simulink library "Garbage"  (R2023a)
settings: Solver=ode45  StartTime=0.0  StopTime=10.0  RelTol=1e-3  EnableMultiTasking=on
undefined symbols (not defined in the file or in its folder): c, ending_column_index, ending_row_index, framebuffer_16x1, framebuffer_2x2, framebuffer_4x4, framebuffer_8x8, HEIGHT, hot, POS_X, POS_Y, starting_column_index, starting_row_index, WIDTH
libraries: Memory_Blocks_Lib x459, simulink x235, simulink_extras x132, dspmtrx3 x116, Bit_Multiplexers_Lib x67, Bit_Demultiplexers_Lib x49, Byte_Multiplexers_Lib x40, Miscellaneous x38, Computer_Library x17, Decoders_Lib x14, commutil2 x12, Arithmetic_Operations_Lib x8
(part 3 of 26)

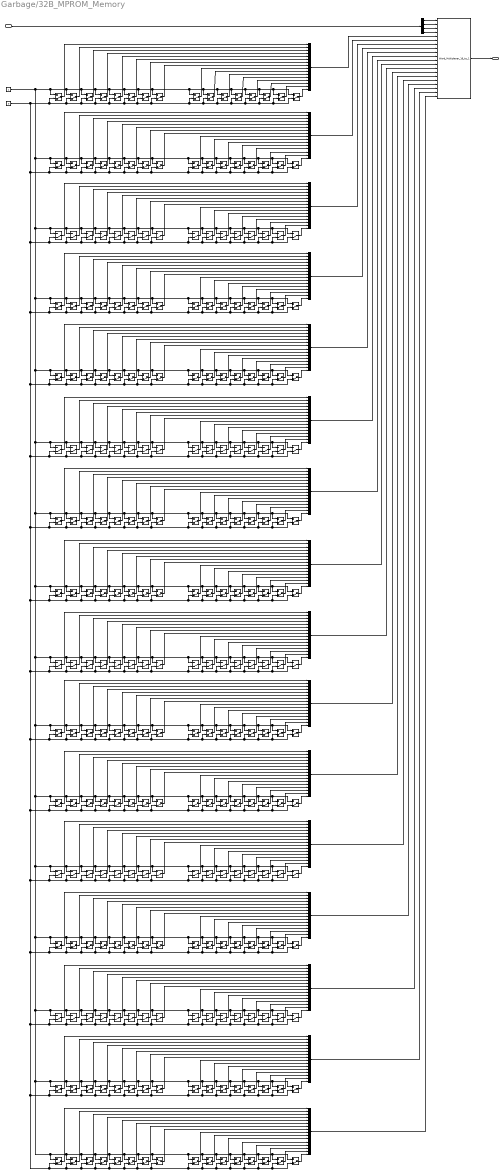
== system Garbage/32B_MPROM_Memory ==
  note: Manually Programmable Read Only Memory (MPROM)
  Addr(2B) : Inport
  Bus Creator{,1..15} (16 alike) : BusCreator  Inputs=16
  Bus Selector : BusSelector  OutputSignals=signal13,signal14,signal15,signal16
  Constant : Constant
  Constant1 : Constant  Value=0
  Manual Switch{,1..156,158..255} (255 alike) : ManualSwitch  CurrentSetting=0
  Manual Switch157 : ManualSwitch
  Word_Multiplexer_16_to_1 : Word_Multiplexers_Lib/Word_Multiplexers_Lib/Word_Multiplexer_16_to_1
  Data(2B) : Outport
  wires:
  Addr(2B)/1 -> Bus Selector/1
  Manual Switch54/1 -> Bus Creator3/16
  Manual Switch59/1 -> Bus Creator3/7
  Manual Switch50/1 -> Bus Creator3/12
  Manual Switch57/1 -> Bus Creator3/5
  Manual Switch53/1 -> Bus Creator3/15
  Manual Switch48/1 -> Bus Creator3/1
  Manual Switch61/1 -> Bus Creator3/9
  Manual Switch52/1 -> Bus Creator3/14
  Manual Switch51/1 -> Bus Creator3/13
  Manual Switch56/1 -> Bus Creator3/4
  Manual Switch49/1 -> Bus Creator3/3
  Manual Switch58/1 -> Bus Creator3/6
  Manual Switch63/1 -> Bus Creator3/11
  Manual Switch62/1 -> Bus Creator3/10
  Manual Switch60/1 -> Bus Creator3/8
  Manual Switch55/1 -> Bus Creator3/2
  Manual Switch14/1 -> Bus Creator/16
  Manual Switch4/1 -> Bus Creator/6
  Manual Switch5/1 -> Bus Creator/7
  Manual Switch3/1 -> Bus Creator/5
  Manual Switch/1 -> Bus Creator/1
  Manual Switch99/1 -> Bus Creator6/13
  Manual Switch98/1 -> Bus Creator6/12
  Manual Switch111/1 -> Bus Creator6/11
  Manual Switch100/1 -> Bus Creator6/14
  Manual Switch110/1 -> Bus Creator6/10
  Manual Switch108/1 -> Bus Creator6/8
  Manual Switch109/1 -> Bus Creator6/9
  Manual Switch102/1 -> Bus Creator6/16
  Manual Switch101/1 -> Bus Creator6/15
  Manual Switch107/1 -> Bus Creator6/7
  Manual Switch104/1 -> Bus Creator6/4
  Manual Switch97/1 -> Bus Creator6/3
  Manual Switch103/1 -> Bus Creator6/2
  Manual Switch96/1 -> Bus Creator6/1
  Manual Switch105/1 -> Bus Creator6/5
  Manual Switch106/1 -> Bus Creator6/6
  Manual Switch85/1 -> Bus Creator5/15
  Manual Switch83/1 -> Bus Creator5/13
  Manual Switch82/1 -> Bus Creator5/12
  Manual Switch86/1 -> Bus Creator5/16
  Manual Switch84/1 -> Bus Creator5/14
  Manual Switch93/1 -> Bus Creator5/9
  Manual Switch91/1 -> Bus Creator5/7
  Manual Switch95/1 -> Bus Creator5/11
  Manual Switch94/1 -> Bus Creator5/10
  Manual Switch92/1 -> Bus Creator5/8
  Manual Switch90/1 -> Bus Creator5/6
  Manual Switch89/1 -> Bus Creator5/5
  Manual Switch88/1 -> Bus Creator5/4
  Manual Switch81/1 -> Bus Creator5/3
  Manual Switch87/1 -> Bus Creator5/2
  Manual Switch80/1 -> Bus Creator5/1
  Manual Switch130/1 -> Bus Creator8/12
  Manual Switch245/1 -> Bus Creator8/11
  Manual Switch234/1 -> Bus Creator8/10
  Manual Switch168/1 -> Bus Creator8/4
  Manual Switch141/1 -> Bus Creator8/13
  Manual Switch128/1 -> Bus Creator8/1
  Manual Switch169/1 -> Bus Creator9/14
  Manual Switch167/1 -> Bus Creator9/13
  Manual Switch166/1 -> Bus Creator9/12
  Manual Switch129/1 -> Bus Creator8/3
  Manual Switch170/1 -> Bus Creator9/15
  Manual Switch162/1 -> Bus Creator8/16
  Manual Switch161/1 -> Bus Creator8/15
  Manual Switch163/1 -> Bus Creator8/2
  Manual Switch171/1 -> Bus Creator9/16
  Manual Switch179/1 -> Bus Creator8/5
  Manual Switch223/1 -> Bus Creator8/9
  Manual Switch212/1 -> Bus Creator8/8
  Manual Switch201/1 -> Bus Creator8/7
  Manual Switch152/1 -> Bus Creator8/14
  Manual Switch190/1 -> Bus Creator8/6
  Manual Switch186/1 -> Bus Creator10/14
  Manual Switch184/1 -> Bus Creator10/12
  Manual Switch197/1 -> Bus Creator10/10
  Manual Switch192/1 -> Bus Creator10/5
  Manual Switch211/1 -> Bus Creator11/7
  Manual Switch209/1 -> Bus Creator11/5
  Manual Switch187/1 -> Bus Creator10/15
  Manual Switch173/1 -> Bus Creator9/4
  Manual Switch195/1 -> Bus Creator10/8
  Manual Switch200/1 -> Bus Creator11/3
  Manual Switch216/1 -> Bus Creator11/11
  Manual Switch199/1 -> Bus Creator11/1
  Manual Switch178/1 -> Bus Creator9/9
  Manual Switch191/1 -> Bus Creator10/4
  Manual Switch214/1 -> Bus Creator11/9
  Manual Switch221/1 -> Bus Creator12/14
  Manual Switch220/1 -> Bus Creator12/13
  Manual Switch232/1 -> Bus Creator12/10
  Manual Switch213/1 -> Bus Creator11/8
  Manual Switch233/1 -> Bus Creator12/11
  Manual Switch231/1 -> Bus Creator12/9
  Manual Switch174/1 -> Bus Creator9/5
  Manual Switch177/1 -> Bus Creator9/8
  Manual Switch189/1 -> Bus Creator10/2
  Manual Switch180/1 -> Bus Creator9/10
  Manual Switch182/1 -> Bus Creator10/1
  Manual Switch203/1 -> Bus Creator11/13
  Manual Switch204/1 -> Bus Creator11/14
  Manual Switch205/1 -> Bus Creator11/15
  Manual Switch181/1 -> Bus Creator9/11
  Manual Switch196/1 -> Bus Creator10/9
  Manual Switch207/1 -> Bus Creator11/2
  Manual Switch222/1 -> Bus Creator12/15
  Manual Switch219/1 -> Bus Creator12/12
  Manual Switch230/1 -> Bus Creator12/8
  Manual Switch198/1 -> Bus Creator10/11
  Manual Switch183/1 -> Bus Creator10/3
  Manual Switch208/1 -> Bus Creator11/4
  Manual Switch194/1 -> Bus Creator10/7
  Manual Switch165/1 -> Bus Creator9/3
  Manual Switch172/1 -> Bus Creator9/2
  Manual Switch229/1 -> Bus Creator12/7
  Manual Switch228/1 -> Bus Creator12/6
  Manual Switch227/1 -> Bus Creator12/5
  Manual Switch202/1 -> Bus Creator11/12
  Manual Switch175/1 -> Bus Creator9/6
  Manual Switch185/1 -> Bus Creator10/13
  Manual Switch215/1 -> Bus Creator11/10
  Manual Switch164/1 -> Bus Creator9/1
  Manual Switch188/1 -> Bus Creator10/16
  Manual Switch210/1 -> Bus Creator11/6
  Manual Switch193/1 -> Bus Creator10/6
  Manual Switch224/1 -> Bus Creator12/16
  Manual Switch206/1 -> Bus Creator11/16
  Manual Switch176/1 -> Bus Creator9/7
  Manual Switch143/1 -> Bus Creator14/11
  Manual Switch236/1 -> Bus Creator13/3
  Manual Switch153/1 -> Bus Creator15/4
  Manual Switch132/1 -> Bus Creator14/15
  Manual Switch140/1 -> Bus Creator14/9
  Manual Switch252/1 -> Bus Creator14/1
  Manual Switch240/1 -> Bus Creator13/15
  Constant/1 -> Manual Switch17/1, Manual Switch24/1, Manual Switch22/1, Manual Switch21/1, Manual Switch20/1, Manual Switch19/1, Manual Switch18/1, Manual Switch31/1, Manual Switch30/1, Manual Switch29/1, Manual Switch28/1, Manual Switch27/1, Manual Switch26/1, Manual Switch25/1, Manual Switch23/1, Manual Switch16/1, Manual Switch33/1, Manual Switch40/1, Manual Switch38/1, Manual Switch37/1, Manual Switch36/1, Manual Switch35/1, Manual Switch34/1, Manual Switch47/1, Manual Switch46/1, Manual Switch45/1, Manual Switch44/1, Manual Switch43/1, Manual Switch42/1, Manual Switch41/1, Manual Switch39/1, Manual Switch32/1, Manual Switch49/1, Manual Switch56/1, Manual Switch54/1, Manual Switch53/1, Manual Switch52/1, Manual Switch51/1, Manual Switch50/1, Manual Switch63/1, Manual Switch62/1, Manual Switch61/1, Manual Switch60/1, Manual Switch59/1, Manual Switch58/1, Manual Switch57/1, Manual Switch55/1, Manual Switch48/1, Manual Switch65/1, Manual Switch72/1, Manual Switch70/1, Manual Switch69/1, Manual Switch68/1, Manual Switch67/1, Manual Switch66/1, Manual Switch79/1, Manual Switch78/1, Manual Switch77/1, Manual Switch76/1, Manual Switch75/1, Manual Switch74/1, Manual Switch73/1, Manual Switch71/1, Manual Switch64/1, Manual Switch81/1, Manual Switch88/1, Manual Switch86/1, Manual Switch85/1, Manual Switch84/1, Manual Switch83/1, Manual Switch82/1, Manual Switch95/1, Manual Switch94/1, Manual Switch93/1, Manual Switch92/1, Manual Switch91/1, Manual Switch90/1, Manual Switch89/1, Manual Switch87/1, Manual Switch80/1, Manual Switch97/1, Manual Switch104/1, Manual Switch102/1, Manual Switch101/1, Manual Switch100/1, Manual Switch99/1, Manual Switch98/1, Manual Switch111/1, Manual Switch110/1, Manual Switch109/1, Manual Switch108/1, Manual Switch107/1, Manual Switch106/1, Manual Switch105/1, Manual Switch103/1, Manual Switch96/1, Manual Switch113/1, Manual Switch120/1, Manual Switch118/1, Manual Switch117/1, Manual Switch116/1, Manual Switch115/1, Manual Switch114/1, Manual Switch127/1, Manual Switch126/1, Manual Switch125/1, Manual Switch124/1, Manual Switch123/1, Manual Switch122/1, Manual Switch121/1, Manual Switch119/1, Manual Switch112/1, Manual Switch129/1, Manual Switch168/1, Manual Switch162/1, Manual Switch161/1, Manual Switch152/1, Manual Switch141/1, Manual Switch130/1, Manual Switch245/1, Manual Switch234/1, Manual Switch223/1, Manual Switch212/1, Manual Switch201/1, Manual Switch190/1, Manual Switch179/1, Manual Switch163/1, Manual Switch128/1, Manual Switch165/1, Manual Switch173/1, Manual Switch171/1, Manual Switch170/1, Manual Switch169/1, Manual Switch167/1, Manual Switch166/1, Manual Switch181/1, Manual Switch180/1, Manual Switch178/1, Manual Switch177/1, Manual Switch176/1, Manual Switch175/1, Manual Switch174/1, Manual Switch172/1, Manual Switch164/1, Manual Switch183/1, Manual Switch191/1, Manual Switch188/1, Manual Switch187/1, Manual Switch186/1, Manual Switch185/1, Manual Switch184/1, Manual Switch198/1, Manual Switch197/1, Manual Switch196/1, Manual Switch195/1, Manual Switch194/1, Manual Switch193/1, Manual Switch192/1, Manual Switch189/1, Manual Switch182/1, Manual Switch200/1, Manual Switch208/1, Manual Switch206/1, Manual Switch205/1, Manual Switch204/1, Manual Switch203/1, Manual Switch202/1, Manual Switch216/1, Manual Switch215/1, Manual Switch214/1, Manual Switch213/1, Manual Switch211/1, Manual Switch210/1, Manual Switch209/1, Manual Switch207/1, Manual Switch199/1, Manual Switch218/1, Manual Switch226/1, Manual Switch224/1, Manual Switch222/1, Manual Switch221/1, Manual Switch220/1, Manual Switch219/1, Manual Switch233/1, Manual Switch232/1, Manual Switch231/1, Manual Switch230/1, Manual Switch229/1, Manual Switch228/1, Manual Switch227/1, Manual Switch225/1, Manual Switch217/1, Manual Switch236/1, Manual Switch243/1, Manual Switch241/1, Manual Switch240/1, Manual Switch239/1, Manual Switch238/1, Manual Switch237/1, Manual Switch251/1, Manual Switch250/1, Manual Switch249/1, Manual Switch248/1, Manual Switch247/1, Manual Switch246/1, Manual Switch244/1, Manual Switch242/1, Manual Switch235/1, Manual Switch253/1, Manual Switch135/1, Manual Switch133/1, Manual Switch132/1, Manual Switch131/1, Manual Switch255/1, Manual Switch254/1, Manual Switch143/1, Manual Switch142/1, Manual Switch140/1, Manual Switch139/1, Manual Switch138/1, Manual Switch137/1, Manual Switch136/1, Manual Switch134/1, Manual Switch252/1, Manual Switch145/1, Manual Switch153/1, Manual Switch150/1, Manual Switch149/1, Manual Switch148/1, Manual Switch147/1, Manual Switch146/1, Manual Switch160/1, Manual Switch159/1, Manual Switch158/1, Manual Switch157/1, Manual Switch156/1, Manual Switch155/1, Manual Switch154/1, Manual Switch151/1, Manual Switch144/1, Manual Switch1/1, Manual Switch2/1, Manual Switch14/1, Manual Switch13/1, Manual Switch12/1, Manual Switch11/1, Manual Switch10/1, Manual Switch9/1, Manual Switch8/1, Manual Switch7/1, Manual Switch6/1, Manual Switch5/1, Manual Switch4/1, Manual Switch3/1, Manual Switch15/1, Manual Switch/1
  Manual Switch254/1 -> Bus Creator14/12
  Manual Switch159/1 -> Bus Creator15/10
  Manual Switch145/1 -> Bus Creator15/3
  Manual Switch151/1 -> Bus Creator15/2
  Manual Switch144/1 -> Bus Creator15/1
  Manual Switch139/1 -> Bus Creator14/8
  Manual Switch138/1 -> Bus Creator14/7
  Manual Switch134/1 -> Bus Creator14/2
  Manual Switch242/1 -> Bus Creator13/2
  Manual Switch239/1 -> Bus Creator13/14
  Manual Switch253/1 -> Bus Creator14/3
  Manual Switch251/1 -> Bus Creator13/11
  Manual Switch149/1 -> Bus Creator15/15
  Manual Switch217/1 -> Bus Creator12/1
  Manual Switch255/1 -> Bus Creator14/13
  Manual Switch249/1 -> Bus Creator13/9
  Manual Switch235/1 -> Bus Creator13/1
  Manual Switch131/1 -> Bus Creator14/14
  Manual Switch225/1 -> Bus Creator12/2
  Manual Switch218/1 -> Bus Creator12/3
  Manual Switch248/1 -> Bus Creator13/8
  Manual Switch136/1 -> Bus Creator14/5
  Manual Switch135/1 -> Bus Creator14/4
  Manual Switch147/1 -> Bus Creator15/13
  Manual Switch146/1 -> Bus Creator15/12
  Manual Switch237/1 -> Bus Creator13/12
  Manual Switch243/1 -> Bus Creator13/4
  Manual Switch250/1 -> Bus Creator13/10
  Manual Switch142/1 -> Bus Creator14/10
  Manual Switch148/1 -> Bus Creator15/14
  Manual Switch160/1 -> Bus Creator15/11
  Manual Switch238/1 -> Bus Creator13/13
  Manual Switch137/1 -> Bus Creator14/6
  Manual Switch158/1 -> Bus Creator15/9
  Manual Switch246/1 -> Bus Creator13/6
  Manual Switch247/1 -> Bus Creator13/7
  Manual Switch157/1 -> Bus Creator15/8
  Manual Switch244/1 -> Bus Creator13/5
  Manual Switch133/1 -> Bus Creator14/16
  Manual Switch150/1 -> Bus Creator15/16
  Manual Switch156/1 -> Bus Creator15/7
  Manual Switch155/1 -> Bus Creator15/6
  Manual Switch154/1 -> Bus Creator15/5
  Manual Switch226/1 -> Bus Creator12/4
  Manual Switch241/1 -> Bus Creator13/16
  Manual Switch13/1 -> Bus Creator/15
  Manual Switch6/1 -> Bus Creator/8
  Manual Switch11/1 -> Bus Creator/13
  Manual Switch9/1 -> Bus Creator/11
  Manual Switch15/1 -> Bus Creator/2
  Manual Switch8/1 -> Bus Creator/10
  Manual Switch10/1 -> Bus Creator/12
  Manual Switch33/1 -> Bus Creator2/3
  Manual Switch35/1 -> Bus Creator2/13
  Manual Switch44/1 -> Bus Creator2/8
  Manual Switch40/1 -> Bus Creator2/4
  Manual Switch43/1 -> Bus Creator2/7
  Manual Switch39/1 -> Bus Creator2/2
  Manual Switch36/1 -> Bus Creator2/14
  Manual Switch47/1 -> Bus Creator2/11
  Manual Switch41/1 -> Bus Creator2/5
  Manual Switch38/1 -> Bus Creator2/16
  Manual Switch34/1 -> Bus Creator2/12
  Manual Switch45/1 -> Bus Creator2/9
  Manual Switch32/1 -> Bus Creator2/1
  Manual Switch42/1 -> Bus Creator2/6
  Manual Switch46/1 -> Bus Creator2/10
  Manual Switch37/1 -> Bus Creator2/15
  Bus Creator2/1 -> Word_Multiplexer_16_to_1/7
  Bus Creator1/1 -> Word_Multiplexer_16_to_1/6
  Bus Creator5/1 -> Word_Multiplexer_16_to_1/10
  Bus Selector/1 -> Word_Multiplexer_16_to_1/1  "<signal13>"
  Bus Creator15/1 -> Word_Multiplexer_16_to_1/20
  Bus Selector/4 -> Word_Multiplexer_16_to_1/4  "<signal16>"
  Bus Creator11/1 -> Word_Multiplexer_16_to_1/16
  Bus Creator14/1 -> Word_Multiplexer_16_to_1/19
  Bus Creator/1 -> Word_Multiplexer_16_to_1/5
  Bus Creator9/1 -> Word_Multiplexer_16_to_1/14
  Bus Creator12/1 -> Word_Multiplexer_16_to_1/17
  Bus Creator13/1 -> Word_Multiplexer_16_to_1/18
  Bus Selector/2 -> Word_Multiplexer_16_to_1/2  "<signal14>"
  Bus Creator4/1 -> Word_Multiplexer_16_to_1/9
  Bus Creator6/1 -> Word_Multiplexer_16_to_1/11
  Bus Selector/3 -> Word_Multiplexer_16_to_1/3  "<signal15>"
  Bus Creator3/1 -> Word_Multiplexer_16_to_1/8
  Bus Creator8/1 -> Word_Multiplexer_16_to_1/13
  Bus Creator10/1 -> Word_Multiplexer_16_to_1/15
  Word_Multiplexer_16_to_1/1 -> Data(2B)/1
  Bus Creator7/1 -> Word_Multiplexer_16_to_1/12
  Manual Switch70/1 -> Bus Creator4/16
  Manual Switch69/1 -> Bus Creator4/15
  Manual Switch79/1 -> Bus Creator4/11
  Manual Switch75/1 -> Bus Creator4/7
  Manual Switch73/1 -> Bus Creator4/5
  Manual Switch68/1 -> Bus Creator4/14
  Manual Switch65/1 -> Bus Creator4/3
  Manual Switch71/1 -> Bus Creator4/2
  Manual Switch74/1 -> Bus Creator4/6
  Manual Switch76/1 -> Bus Creator4/8
  Manual Switch64/1 -> Bus Creator4/1
  Manual Switch72/1 -> Bus Creator4/4
  Manual Switch78/1 -> Bus Creator4/10
  Manual Switch66/1 -> Bus Creator4/12
  Manual Switch77/1 -> Bus Creator4/9
  Manual Switch67/1 -> Bus Creator4/13
  Manual Switch127/1 -> Bus Creator7/11
  Manual Switch125/1 -> Bus Creator7/9
  Manual Switch119/1 -> Bus Creator7/2
  Manual Switch122/1 -> Bus Creator7/6
  Manual Switch112/1 -> Bus Creator7/1
  Manual Switch121/1 -> Bus Creator7/5
  Manual Switch120/1 -> Bus Creator7/4
  Manual Switch113/1 -> Bus Creator7/3
  Manual Switch124/1 -> Bus Creator7/8
  Manual Switch126/1 -> Bus Creator7/10
  Manual Switch123/1 -> Bus Creator7/7
  Manual Switch2/1 -> Bus Creator/4
  Manual Switch12/1 -> Bus Creator/14
  Manual Switch115/1 -> Bus Creator7/13
  Manual Switch114/1 -> Bus Creator7/12
  Manual Switch7/1 -> Bus Creator/9
  Manual Switch1/1 -> Bus Creator/3
  Manual Switch118/1 -> Bus Creator7/16
  Manual Switch116/1 -> Bus Creator7/14
  Manual Switch117/1 -> Bus Creator7/15
  Manual Switch24/1 -> Bus Creator1/4
  Manual Switch22/1 -> Bus Creator1/16
  Manual Switch30/1 -> Bus Creator1/10
  Manual Switch26/1 -> Bus Creator1/6
  Manual Switch20/1 -> Bus Creator1/14
  Manual Switch19/1 -> Bus Creator1/13
  Constant1/1 -> Manual Switch23/2, Manual Switch17/2, Manual Switch25/2, Manual Switch26/2, Manual Switch22/2, Manual Switch21/2, Manual Switch20/2, Manual Switch19/2, Manual Switch18/2, Manual Switch31/2, Manual Switch30/2, Manual Switch29/2, Manual Switch28/2, Manual Switch27/2, Manual Switch24/2, Manual Switch16/2, Manual Switch39/2, Manual Switch33/2, Manual Switch41/2, Manual Switch42/2, Manual Switch38/2, Manual Switch37/2, Manual Switch36/2, Manual Switch35/2, Manual Switch34/2, Manual Switch47/2, Manual Switch46/2, Manual Switch45/2, Manual Switch44/2, Manual Switch43/2, Manual Switch40/2, Manual Switch32/2, Manual Switch55/2, Manual Switch49/2, Manual Switch57/2, Manual Switch58/2, Manual Switch54/2, Manual Switch53/2, Manual Switch52/2, Manual Switch51/2, Manual Switch50/2, Manual Switch63/2, Manual Switch62/2, Manual Switch61/2, Manual Switch60/2, Manual Switch59/2, Manual Switch56/2, Manual Switch48/2, Manual Switch71/2, Manual Switch65/2, Manual Switch73/2, Manual Switch74/2, Manual Switch70/2, Manual Switch69/2, Manual Switch68/2, Manual Switch67/2, Manual Switch66/2, Manual Switch79/2, Manual Switch78/2, Manual Switch77/2, Manual Switch76/2, Manual Switch75/2, Manual Switch72/2, Manual Switch64/2, Manual Switch103/2, Manual Switch97/2, Manual Switch105/2, Manual Switch106/2, Manual Switch102/2, Manual Switch101/2, Manual Switch100/2, Manual Switch99/2, Manual Switch98/2, Manual Switch111/2, Manual Switch110/2, Manual Switch109/2, Manual Switch108/2, Manual Switch107/2, Manual Switch104/2, Manual Switch96/2, Manual Switch119/2, Manual Switch113/2, Manual Switch121/2, Manual Switch122/2, Manual Switch118/2, Manual Switch117/2, Manual Switch116/2, Manual Switch115/2, Manual Switch114/2, Manual Switch127/2, Manual Switch126/2, Manual Switch125/2, Manual Switch124/2, Manual Switch123/2, Manual Switch120/2, Manual Switch112/2, Manual Switch163/2, Manual Switch129/2, Manual Switch179/2, Manual Switch190/2, Manual Switch162/2, Manual Switch161/2, Manual Switch152/2, Manual Switch141/2, Manual Switch130/2, Manual Switch245/2, Manual Switch234/2, Manual Switch223/2, Manual Switch212/2, Manual Switch201/2, Manual Switch168/2, Manual Switch128/2, Manual Switch172/2, Manual Switch165/2, Manual Switch174/2, Manual Switch175/2, Manual Switch171/2, Manual Switch170/2, Manual Switch169/2, Manual Switch167/2, Manual Switch166/2, Manual Switch181/2, Manual Switch180/2, Manual Switch178/2, Manual Switch177/2, Manual Switch176/2, Manual Switch173/2, Manual Switch164/2, Manual Switch189/2, Manual Switch183/2, Manual Switch192/2, Manual Switch193/2, Manual Switch188/2, Manual Switch187/2, Manual Switch186/2, Manual Switch185/2, Manual Switch184/2, Manual Switch198/2, Manual Switch197/2, Manual Switch196/2, Manual Switch195/2, Manual Switch194/2, Manual Switch191/2, Manual Switch182/2, Manual Switch207/2, Manual Switch200/2, Manual Switch209/2, Manual Switch210/2, Manual Switch206/2, Manual Switch205/2, Manual Switch204/2, Manual Switch203/2, Manual Switch202/2, Manual Switch216/2, Manual Switch215/2, Manual Switch214/2, Manual Switch213/2, Manual Switch211/2, Manual Switch208/2, Manual Switch199/2, Manual Switch225/2, Manual Switch218/2, Manual Switch227/2, Manual Switch228/2, Manual Switch224/2, Manual Switch222/2, Manual Switch221/2, Manual Switch220/2, Manual Switch219/2, Manual Switch233/2, Manual Switch232/2, Manual Switch231/2, Manual Switch230/2, Manual Switch229/2, Manual Switch226/2, Manual Switch217/2, Manual Switch242/2, Manual Switch236/2, Manual Switch244/2, Manual Switch246/2, Manual Switch241/2, Manual Switch240/2, Manual Switch239/2, Manual Switch238/2, Manual Switch237/2, Manual Switch251/2, Manual Switch250/2, Manual Switch249/2, Manual Switch248/2, Manual Switch247/2, Manual Switch243/2, Manual Switch235/2, Manual Switch134/2, Manual Switch253/2, Manual Switch136/2, Manual Switch137/2, Manual Switch133/2, Manual Switch132/2, Manual Switch131/2, Manual Switch255/2, Manual Switch254/2, Manual Switch143/2, Manual Switch142/2, Manual Switch140/2, Manual Switch139/2, Manual Switch138/2, Manual Switch135/2, Manual Switch252/2, Manual Switch151/2, Manual Switch145/2, Manual Switch154/2, Manual Switch155/2, Manual Switch150/2, Manual Switch149/2, Manual Switch148/2, Manual Switch147/2, Manual Switch146/2, Manual Switch160/2, Manual Switch159/2, Manual Switch158/2, Manual Switch157/2, Manual Switch156/2, Manual Switch153/2, Manual Switch144/2, Manual Switch87/2, Manual Switch81/2, Manual Switch89/2, Manual Switch90/2, Manual Switch86/2, Manual Switch85/2, Manual Switch84/2, Manual Switch83/2, Manual Switch82/2, Manual Switch95/2, Manual Switch94/2, Manual Switch93/2, Manual Switch92/2, Manual Switch91/2, Manual Switch88/2, Manual Switch80/2, Manual Switch15/2, Manual Switch1/2, Manual Switch3/2, Manual Switch4/2, Manual Switch14/2, Manual Switch13/2, Manual Switch12/2, Manual Switch11/2, Manual Switch10/2, Manual Switch9/2, Manual Switch8/2, Manual Switch7/2, Manual Switch6/2, Manual Switch5/2, Manual Switch2/2, Manual Switch/2
  Manual Switch27/1 -> Bus Creator1/7
  Manual Switch21/1 -> Bus Creator1/15
  Manual Switch25/1 -> Bus Creator1/5
  Manual Switch17/1 -> Bus Creator1/3
  Manual Switch31/1 -> Bus Creator1/11
  Manual Switch29/1 -> Bus Creator1/9
  Manual Switch28/1 -> Bus Creator1/8
  Manual Switch23/1 -> Bus Creator1/2
  Manual Switch16/1 -> Bus Creator1/1
  Manual Switch18/1 -> Bus Creator1/12

== system Garbage/32B_RAM_Memory  ==
  Addr(2B) : Inport
  Data(2B) : Inport  Port=2
  Write(1b) : Inport  Port=3
  CLK : Inport  Port=4  OutDataTypeStr=double
  4_to_16_Decoder : Decoders_Lib/Decoders_Lib/Decoder_4_to_16
  Bit to Integer Converter{1..16} (16 alike) : simulink/Logic and Bit Operations/Bit to Integer Converter  nbits=16  bitOrder=MSB first  signedOutputValues=Unsigned  outDtype=uint16  outDtypeSigned=Inherit via internal rule
      settings: LibrarySourceBlock=|
      | commutil2/Bit to Integer
      | Converter
  Bus Selector1 : BusSelector  OutputSignals=signal13,signal14,signal15,signal16
  Word_{0..15} (16 alike) : Memory_Blocks_Lib/Memory_Blocks_Lib/Word  SystemSampleTime=-1  VariantActivationTime=update diagram  Latency=0  AutoFrameSizeCalculation=off
  Word_Multiplexer_16_to_1 : Word_Multiplexers_Lib/Word_Multiplexers_Lib/Word_Multiplexer_16_to_1
  DataOut(2B) : Outport
  tag scope: RAM_16=global, RAM_17=global, RAM_18=global, RAM_19=global, RAM_20=global, RAM_21=global, RAM_22=global, RAM_23=global, RAM_24=global, RAM_25=global, RAM_26=global, RAM_27=global, RAM_28=global, RAM_29=global, RAM_30=global, RAM_31=global
  wires:
  Bus Selector1/4 -> 4_to_16_Decoder/4, Word_Multiplexer_16_to_1/4  "<signal16>"
  Bus Selector1/3 -> 4_to_16_Decoder/3, Word_Multiplexer_16_to_1/3  "<signal15>"
  Bus Selector1/2 -> 4_to_16_Decoder/2, Word_Multiplexer_16_to_1/2  "<signal14>"
  CLK/1 -> Word_0/4, Word_1/4, Word_2/4, Word_3/4, Word_4/4, Word_5/4, Word_6/4, Word_7/4, Word_8/4, Word_9/4, Word_10/4, Word_11/4, Word_12/4, Word_13/4, Word_14/4, Word_15/4
  Data(2B)/1 -> Word_11/3, Word_10/3, Word_0/3, Word_1/3, Word_2/3, Word_3/3, Word_4/3, Word_5/3, Word_6/3, Word_7/3, Word_8/3, Word_9/3, Word_12/3, Word_13/3, Word_14/3, Word_15/3
  Write(1b)/1 -> Word_0/2, Word_1/2, Word_2/2, Word_3/2, Word_4/2, Word_5/2, Word_6/2, Word_7/2, Word_8/2, Word_9/2, Word_10/2, Word_11/2, Word_12/2, Word_13/2, Word_14/2, Word_15/2
  4_to_16_Decoder/1 -> Word_15/1
  Bus Selector1/1 -> 4_to_16_Decoder/1, Word_Multiplexer_16_to_1/1  "<signal13>"
  4_to_16_Decoder/2 -> Word_14/1
  4_to_16_Decoder/3 -> Word_13/1
  4_to_16_Decoder/4 -> Word_12/1
  4_to_16_Decoder/5 -> Word_11/1
  4_to_16_Decoder/6 -> Word_10/1
  4_to_16_Decoder/7 -> Word_9/1
  4_to_16_Decoder/8 -> Word_8/1
  4_to_16_Decoder/9 -> Word_7/1
  4_to_16_Decoder/10 -> Word_6/1
  4_to_16_Decoder/11 -> Word_5/1
  4_to_16_Decoder/12 -> Word_4/1
  4_to_16_Decoder/13 -> Word_3/1
  4_to_16_Decoder/14 -> Word_2/1
  4_to_16_Decoder/15 -> Word_1/1
  4_to_16_Decoder/16 -> Word_0/1
  Word_15/1 -> Bit to Integer Converter16/1, Word_Multiplexer_16_to_1/5
  Word_14/1 -> Bit to Integer Converter14/1, Word_Multiplexer_16_to_1/6
  Word_13/1 -> Bit to Integer Converter13/1, Word_Multiplexer_16_to_1/7
  Word_12/1 -> Bit to Integer Converter12/1, Word_Multiplexer_16_to_1/8
  Word_11/1 -> Bit to Integer Converter11/1, Word_Multiplexer_16_to_1/9
  Word_10/1 -> Bit to Integer Converter10/1, Word_Multiplexer_16_to_1/10
  Word_9/1 -> Bit to Integer Converter9/1, Word_Multiplexer_16_to_1/11
  Word_8/1 -> Bit to Integer Converter8/1, Word_Multiplexer_16_to_1/12
  Word_7/1 -> Bit to Integer Converter7/1, Word_Multiplexer_16_to_1/13
  Word_6/1 -> Bit to Integer Converter6/1, Word_Multiplexer_16_to_1/14
  Word_5/1 -> Bit to Integer Converter5/1, Word_Multiplexer_16_to_1/15
  Word_4/1 -> Bit to Integer Converter4/1, Word_Multiplexer_16_to_1/16
  Word_3/1 -> Bit to Integer Converter3/1, Word_Multiplexer_16_to_1/17
  Word_2/1 -> Bit to Integer Converter2/1, Word_Multiplexer_16_to_1/18
  Word_1/1 -> Bit to Integer Converter1/1, Word_Multiplexer_16_to_1/19
  Word_0/1 -> Bit to Integer Converter15/1, Word_Multiplexer_16_to_1/20
  Word_Multiplexer_16_to_1/1 -> DataOut(2B)/1
  Addr(2B)/1 -> Bus Selector1/1
  Bit to Integer Converter15/1 -> [RAM_16]
  Bit to Integer Converter1/1 -> [RAM_17]
  Bit to Integer Converter2/1 -> [RAM_18]
  Bit to Integer Converter3/1 -> [RAM_19]
  Bit to Integer Converter4/1 -> [RAM_20]
  Bit to Integer Converter5/1 -> [RAM_21]
  Bit to Integer Converter6/1 -> [RAM_22]
  Bit to Integer Converter7/1 -> [RAM_23]
  Bit to Integer Converter8/1 -> [RAM_24]
  Bit to Integer Converter9/1 -> [RAM_25]
  Bit to Integer Converter10/1 -> [RAM_26]
  Bit to Integer Converter11/1 -> [RAM_27]
  Bit to Integer Converter12/1 -> [RAM_28]
  Bit to Integer Converter13/1 -> [RAM_29]
  Bit to Integer Converter14/1 -> [RAM_30]
  Bit to Integer Converter16/1 -> [RAM_31]

== system Garbage/32B_VRAM ==
  Addr(2B) : Inport
  Data(2B) : Inport  Port=2
  Write(1b) : Inport  Port=3
  CLK : Inport  Port=4  OutDataTypeStr=double
  4_to_16_Decoder : Decoders_Lib/Decoders_Lib/Decoder_4_to_16
  Bit to Integer Converter{1..32} (32 alike) : simulink/Logic and Bit Operations/Bit to Integer Converter  nbits=16  bitOrder=MSB first  signedOutputValues=Unsigned  outDtype=uint16  outDtypeSigned=Inherit via internal rule
      settings: LibrarySourceBlock=|
      | commutil2/Bit to Integer
      | Converter
  Bus Selector1 : BusSelector  OutputSignals=signal13,signal14,signal15,signal16
  Vector Concatenate : Concatenate  NumInputs=16
  Word_{0..15} (16 alike) : Memory_Blocks_Lib/Memory_Blocks_Lib/Word  SystemSampleTime=-1  VariantActivationTime=update diagram  Latency=0  AutoFrameSizeCalculation=off
  Word_Multiplexer_16_to_1 : Word_Multiplexers_Lib/Word_Multiplexers_Lib/Word_Multiplexer_16_to_1
  DataOut(2B) : Outport
  Video(16x2B) : Outport  Port=2  OutDataTypeStr=uint16  PortDimensions=16
  tag scope: VRAM_00=global, VRAM_01=global, VRAM_02=global, VRAM_03=global, VRAM_04=global, VRAM_05=global, VRAM_06=global, VRAM_07=global, VRAM_08=global, VRAM_09=global, VRAM_10=global, VRAM_11=global, VRAM_12=global, VRAM_13=global, VRAM_14=global, VRAM_15=global
  wires:
  Bus Selector1/4 -> 4_to_16_Decoder/4, Word_Multiplexer_16_to_1/4  "<signal16>"
  Bus Selector1/3 -> 4_to_16_Decoder/3, Word_Multiplexer_16_to_1/3  "<signal15>"
  Bus Selector1/2 -> 4_to_16_Decoder/2, Word_Multiplexer_16_to_1/2  "<signal14>"
  CLK/1 -> Word_0/4, Word_1/4, Word_2/4, Word_3/4, Word_4/4, Word_5/4, Word_6/4, Word_7/4, Word_8/4, Word_9/4, Word_10/4, Word_11/4, Word_12/4, Word_13/4, Word_14/4, Word_15/4
  Data(2B)/1 -> Word_11/3, Word_10/3, Word_0/3, Word_1/3, Word_2/3, Word_3/3, Word_4/3, Word_5/3, Word_6/3, Word_7/3, Word_8/3, Word_9/3, Word_12/3, Word_13/3, Word_14/3, Word_15/3
  Write(1b)/1 -> Word_0/2, Word_1/2, Word_2/2, Word_3/2, Word_4/2, Word_5/2, Word_6/2, Word_7/2, Word_8/2, Word_9/2, Word_10/2, Word_11/2, Word_12/2, Word_13/2, Word_14/2, Word_15/2
  4_to_16_Decoder/1 -> Word_15/1
  Bus Selector1/1 -> 4_to_16_Decoder/1, Word_Multiplexer_16_to_1/1  "<signal13>"
  4_to_16_Decoder/2 -> Word_14/1
  4_to_16_Decoder/3 -> Word_13/1
  4_to_16_Decoder/4 -> Word_12/1
  4_to_16_Decoder/5 -> Word_11/1
  4_to_16_Decoder/6 -> Word_10/1
  4_to_16_Decoder/7 -> Word_9/1
  4_to_16_Decoder/8 -> Word_8/1
  4_to_16_Decoder/9 -> Word_7/1
  4_to_16_Decoder/10 -> Word_6/1
  4_to_16_Decoder/11 -> Word_5/1
  4_to_16_Decoder/12 -> Word_4/1
  4_to_16_Decoder/13 -> Word_3/1
  4_to_16_Decoder/14 -> Word_2/1
  4_to_16_Decoder/15 -> Word_1/1
  4_to_16_Decoder/16 -> Word_0/1
  Word_Multiplexer_16_to_1/1 -> DataOut(2B)/1
  Addr(2B)/1 -> Bus Selector1/1
  Bit to Integer Converter15/1 -> [VRAM_00]
  Bit to Integer Converter1/1 -> [VRAM_01]
  Bit to Integer Converter2/1 -> [VRAM_02]
  Bit to Integer Converter3/1 -> [VRAM_03]
  Bit to Integer Converter4/1 -> [VRAM_04]
  Bit to Integer Converter5/1 -> [VRAM_05]
  Bit to Integer Converter6/1 -> [VRAM_06]
  Bit to Integer Converter7/1 -> [VRAM_07]
  Bit to Integer Converter8/1 -> [VRAM_08]
  Bit to Integer Converter9/1 -> [VRAM_09]
  Bit to Integer Converter10/1 -> [VRAM_10]
  Bit to Integer Converter11/1 -> [VRAM_11]
  Bit to Integer Converter12/1 -> [VRAM_12]
  Bit to Integer Converter13/1 -> [VRAM_13]
  Bit to Integer Converter14/1 -> [VRAM_14]
  Bit to Integer Converter16/1 -> [VRAM_15]
  Word_5/1 -> Bit to Integer Converter5/1, Bit to Integer Converter27/1, Word_Multiplexer_16_to_1/15
  Word_1/1 -> Bit to Integer Converter1/1, Bit to Integer Converter31/1, Word_Multiplexer_16_to_1/19
  Word_7/1 -> Bit to Integer Converter7/1, Bit to Integer Converter25/1, Word_Multiplexer_16_to_1/13
  Word_8/1 -> Bit to Integer Converter8/1, Bit to Integer Converter24/1, Word_Multiplexer_16_to_1/12
  Word_9/1 -> Bit to Integer Converter9/1, Bit to Integer Converter23/1, Word_Multiplexer_16_to_1/11
  Word_4/1 -> Bit to Integer Converter4/1, Bit to Integer Converter28/1, Word_Multiplexer_16_to_1/16
  Word_14/1 -> Bit to Integer Converter14/1, Bit to Integer Converter18/1, Word_Multiplexer_16_to_1/6
  Word_10/1 -> Bit to Integer Converter10/1, Bit to Integer Converter22/1, Word_Multiplexer_16_to_1/10
  Word_3/1 -> Bit to Integer Converter3/1, Bit to Integer Converter29/1, Word_Multiplexer_16_to_1/17
  Word_12/1 -> Bit to Integer Converter12/1, Bit to Integer Converter20/1, Word_Multiplexer_16_to_1/8
  Word_0/1 -> Bit to Integer Converter15/1, Bit to Integer Converter32/1, Word_Multiplexer_16_to_1/20
  Word_2/1 -> Bit to Integer Converter2/1, Bit to Integer Converter30/1, Word_Multiplexer_16_to_1/18
  Word_15/1 -> Bit to Integer Converter16/1, Bit to Integer Converter17/1, Word_Multiplexer_16_to_1/5
  Word_11/1 -> Bit to Integer Converter11/1, Bit to Integer Converter21/1, Word_Multiplexer_16_to_1/9
  Word_6/1 -> Bit to Integer Converter6/1, Bit to Integer Converter26/1, Word_Multiplexer_16_to_1/14
  Word_13/1 -> Bit to Integer Converter13/1, Bit to Integer Converter19/1, Word_Multiplexer_16_to_1/7
  Vector Concatenate/1 -> Video(16x2B)/1
  Bit to Integer Converter17/1 -> Vector Concatenate/16
  Bit to Integer Converter18/1 -> Vector Concatenate/15
  Bit to Integer Converter19/1 -> Vector Concatenate/14
  Bit to Integer Converter20/1 -> Vector Concatenate/13
  Bit to Integer Converter21/1 -> Vector Concatenate/12
  Bit to Integer Converter22/1 -> Vector Concatenate/11
  Bit to Integer Converter23/1 -> Vector Concatenate/10
  Bit to Integer Converter24/1 -> Vector Concatenate/9
  Bit to Integer Converter25/1 -> Vector Concatenate/8
  Bit to Integer Converter26/1 -> Vector Concatenate/7
  Bit to Integer Converter27/1 -> Vector Concatenate/6
  Bit to Integer Converter28/1 -> Vector Concatenate/5
  Bit to Integer Converter29/1 -> Vector Concatenate/4
  Bit to Integer Converter30/1 -> Vector Concatenate/3
  Bit to Integer Converter31/1 -> Vector Concatenate/2
  Bit to Integer Converter32/1 -> Vector Concatenate/1

== system Garbage/32B_VRAM  ==
  Addr(2B) : Inport
  Data(2B) : Inport  Port=2
  Write(1b) : Inport  Port=3
  CLK : Inport  Port=4  OutDataTypeStr=double
  4_to_16_Decoder : Decoders_Lib/Decoders_Lib/Decoder_4_to_16
  Bit to Integer Converter{1..32} (32 alike) : simulink/Logic and Bit Operations/Bit to Integer Converter  nbits=16  bitOrder=MSB first  signedOutputValues=Unsigned  outDtype=uint16  outDtypeSigned=Inherit via internal rule
      settings: LibrarySourceBlock=|
      | commutil2/Bit to Integer
      | Converter
  Bus Selector1 : BusSelector  OutputSignals=signal13,signal14,signal15,signal16
  Vector Concatenate : Concatenate  NumInputs=16
  Word_{0..15} (16 alike) : Memory_Blocks_Lib/Memory_Blocks_Lib/Word  SystemSampleTime=-1  VariantActivationTime=update diagram  Latency=0  AutoFrameSizeCalculation=off
  Word_Multiplexer_16_to_1 : Word_Multiplexers_Lib/Word_Multiplexers_Lib/Word_Multiplexer_16_to_1
  DataOut(2B) : Outport
  Video(16x2B) : Outport  Port=2  OutDataTypeStr=uint16  PortDimensions=16
  tag scope: VRAM_16=global, VRAM_17=global, VRAM_18=global, VRAM_19=global, VRAM_20=global, VRAM_21=global, VRAM_22=global, VRAM_23=global, VRAM_24=global, VRAM_25=global, VRAM_26=global, VRAM_27=global, VRAM_28=global, VRAM_29=global, VRAM_30=global, VRAM_31=global
  wires:
  Bus Selector1/4 -> 4_to_16_Decoder/4, Word_Multiplexer_16_to_1/4  "<signal16>"
  Bus Selector1/3 -> 4_to_16_Decoder/3, Word_Multiplexer_16_to_1/3  "<signal15>"
  Bus Selector1/2 -> 4_to_16_Decoder/2, Word_Multiplexer_16_to_1/2  "<signal14>"
  CLK/1 -> Word_0/4, Word_1/4, Word_2/4, Word_3/4, Word_4/4, Word_5/4, Word_6/4, Word_7/4, Word_8/4, Word_9/4, Word_10/4, Word_11/4, Word_12/4, Word_13/4, Word_14/4, Word_15/4
  Data(2B)/1 -> Word_11/3, Word_10/3, Word_0/3, Word_1/3, Word_2/3, Word_3/3, Word_4/3, Word_5/3, Word_6/3, Word_7/3, Word_8/3, Word_9/3, Word_12/3, Word_13/3, Word_14/3, Word_15/3
  Write(1b)/1 -> Word_0/2, Word_1/2, Word_2/2, Word_3/2, Word_4/2, Word_5/2, Word_6/2, Word_7/2, Word_8/2, Word_9/2, Word_10/2, Word_11/2, Word_12/2, Word_13/2, Word_14/2, Word_15/2
  4_to_16_Decoder/1 -> Word_15/1
  Bus Selector1/1 -> 4_to_16_Decoder/1, Word_Multiplexer_16_to_1/1  "<signal13>"
  4_to_16_Decoder/2 -> Word_14/1
  4_to_16_Decoder/3 -> Word_13/1
  4_to_16_Decoder/4 -> Word_12/1
  4_to_16_Decoder/5 -> Word_11/1
  4_to_16_Decoder/6 -> Word_10/1
  4_to_16_Decoder/7 -> Word_9/1
  4_to_16_Decoder/8 -> Word_8/1
  4_to_16_Decoder/9 -> Word_7/1
  4_to_16_Decoder/10 -> Word_6/1
  4_to_16_Decoder/11 -> Word_5/1
  4_to_16_Decoder/12 -> Word_4/1
  4_to_16_Decoder/13 -> Word_3/1
  4_to_16_Decoder/14 -> Word_2/1
  4_to_16_Decoder/15 -> Word_1/1
  4_to_16_Decoder/16 -> Word_0/1
  Word_Multiplexer_16_to_1/1 -> DataOut(2B)/1
  Addr(2B)/1 -> Bus Selector1/1
  Bit to Integer Converter15/1 -> [VRAM_16]
  Bit to Integer Converter1/1 -> [VRAM_17]
  Bit to Integer Converter2/1 -> [VRAM_18]
  Bit to Integer Converter3/1 -> [VRAM_19]
  Bit to Integer Converter4/1 -> [VRAM_20]
  Bit to Integer Converter5/1 -> [VRAM_21]
  Bit to Integer Converter6/1 -> [VRAM_22]
  Bit to Integer Converter7/1 -> [VRAM_23]
  Bit to Integer Converter8/1 -> [VRAM_24]
  Bit to Integer Converter9/1 -> [VRAM_25]
  Bit to Integer Converter10/1 -> [VRAM_26]
  Bit to Integer Converter11/1 -> [VRAM_27]
  Bit to Integer Converter12/1 -> [VRAM_28]
  Bit to Integer Converter13/1 -> [VRAM_29]
  Bit to Integer Converter14/1 -> [VRAM_30]
  Bit to Integer Converter16/1 -> [VRAM_31]
  Word_5/1 -> Bit to Integer Converter5/1, Bit to Integer Converter27/1, Word_Multiplexer_16_to_1/15
  Word_1/1 -> Bit to Integer Converter1/1, Bit to Integer Converter31/1, Word_Multiplexer_16_to_1/19
  Word_7/1 -> Bit to Integer Converter7/1, Bit to Integer Converter25/1, Word_Multiplexer_16_to_1/13
  Word_8/1 -> Bit to Integer Converter8/1, Bit to Integer Converter24/1, Word_Multiplexer_16_to_1/12
  Word_9/1 -> Bit to Integer Converter9/1, Bit to Integer Converter23/1, Word_Multiplexer_16_to_1/11
  Word_4/1 -> Bit to Integer Converter4/1, Bit to Integer Converter28/1, Word_Multiplexer_16_to_1/16
  Word_14/1 -> Bit to Integer Converter14/1, Bit to Integer Converter18/1, Word_Multiplexer_16_to_1/6
  Word_10/1 -> Bit to Integer Converter10/1, Bit to Integer Converter22/1, Word_Multiplexer_16_to_1/10
  Word_3/1 -> Bit to Integer Converter3/1, Bit to Integer Converter29/1, Word_Multiplexer_16_to_1/17
  Word_12/1 -> Bit to Integer Converter12/1, Bit to Integer Converter20/1, Word_Multiplexer_16_to_1/8
  Word_0/1 -> Bit to Integer Converter15/1, Bit to Integer Converter32/1, Word_Multiplexer_16_to_1/20
  Word_2/1 -> Bit to Integer Converter2/1, Bit to Integer Converter30/1, Word_Multiplexer_16_to_1/18
  Word_15/1 -> Bit to Integer Converter16/1, Bit to Integer Converter17/1, Word_Multiplexer_16_to_1/5
  Word_11/1 -> Bit to Integer Converter11/1, Bit to Integer Converter21/1, Word_Multiplexer_16_to_1/9
  Word_6/1 -> Bit to Integer Converter6/1, Bit to Integer Converter26/1, Word_Multiplexer_16_to_1/14
  Word_13/1 -> Bit to Integer Converter13/1, Bit to Integer Converter19/1, Word_Multiplexer_16_to_1/7
  Vector Concatenate/1 -> Video(16x2B)/1
  Bit to Integer Converter17/1 -> Vector Concatenate/16
  Bit to Integer Converter18/1 -> Vector Concatenate/15
  Bit to Integer Converter19/1 -> Vector Concatenate/14
  Bit to Integer Converter20/1 -> Vector Concatenate/13
  Bit to Integer Converter21/1 -> Vector Concatenate/12
  Bit to Integer Converter22/1 -> Vector Concatenate/11
  Bit to Integer Converter23/1 -> Vector Concatenate/10
  Bit to Integer Converter24/1 -> Vector Concatenate/9
  Bit to Integer Converter25/1 -> Vector Concatenate/8
  Bit to Integer Converter26/1 -> Vector Concatenate/7
  Bit to Integer Converter27/1 -> Vector Concatenate/6
  Bit to Integer Converter28/1 -> Vector Concatenate/5
  Bit to Integer Converter29/1 -> Vector Concatenate/4
  Bit to Integer Converter30/1 -> Vector Concatenate/3
  Bit to Integer Converter31/1 -> Vector Concatenate/2
  Bit to Integer Converter32/1 -> Vector Concatenate/1

== system Garbage/<0x0000..0x0FFF> ==
  note: Address_Select
  Addr(2B) : Inport
  1 : Constant  OutDataTypeStr=boolean
  Bus Selector6 : BusSelector  OutputSignals=b15,b14,b13,b12,b11,b10,b09,b08,b07,b06,b05,b04,b03,b02,b01,b00
  Logical Operator{10..12,17} (4 alike) : Logic  Operator=NOT  Inputs=1  IconShape=distinctive  AllPortsSameDT=off  OutDataTypeStr=boolean
  Logical Operator9 : Logic  Inputs=16  IconShape=distinctive  AllPortsSameDT=off  OutDataTypeStr=boolean
  Terminator{1..12} (12 alike) : Terminator
  Select(1b) : Outport
  wires:
  Logical Operator9/1 -> Select(1b)/1
  Addr(2B)/1 -> Bus Selector6/1
  Bus Selector6/3 -> Logical Operator12/1  "<b13>"
  Bus Selector6/1 -> Logical Operator10/1  "<b15>"
  Bus Selector6/2 -> Logical Operator11/1  "<b14>"
  Bus Selector6/4 -> Logical Operator17/1  "<b12>"
  Logical Operator10/1 -> Logical Operator9/1
  Logical Operator11/1 -> Logical Operator9/2
  Logical Operator12/1 -> Logical Operator9/3
  Logical Operator17/1 -> Logical Operator9/4
  Bus Selector6/16 -> Terminator12/1  "<b00>"
  Bus Selector6/5 -> Terminator9/1  "<b11>"
  Bus Selector6/6 -> Terminator8/1  "<b10>"
  Bus Selector6/7 -> Terminator7/1  "<b09>"
  Bus Selector6/8 -> Terminator6/1  "<b08>"
  Bus Selector6/9 -> Terminator5/1  "<b07>"
  Bus Selector6/10 -> Terminator4/1  "<b06>"
  Bus Selector6/11 -> Terminator3/1  "<b05>"
  Bus Selector6/12 -> Terminator1/1  "<b04>"
  Bus Selector6/13 -> Terminator2/1  "<b03>"
  Bus Selector6/14 -> Terminator10/1  "<b02>"
  Bus Selector6/15 -> Terminator11/1  "<b01>"
  1/1 -> Logical Operator9/16, Logical Operator9/15, Logical Operator9/14, Logical Operator9/13, Logical Operator9/12, Logical Operator9/11, Logical Operator9/10, Logical Operator9/9, Logical Operator9/8, Logical Operator9/7, Logical Operator9/6, Logical Operator9/5

== system Garbage/<0x2000..0x23FF> ==
  note: Address_Select
  Addr(2B) : Inport
  1 : Constant  OutDataTypeStr=boolean
  Bus Selector1 : BusSelector  OutputSignals=b15,b14,b13,b12,b11,b10,b09,b08,b07,b06,b05,b04,b03,b02,b01,b00
  Logical Operator{1..2,10..12} (5 alike) : Logic  Operator=NOT  Inputs=1  IconShape=distinctive  AllPortsSameDT=off  OutDataTypeStr=boolean
  Logical Operator13 : Logic  Inputs=16  IconShape=distinctive  AllPortsSameDT=off  OutDataTypeStr=boolean
  Terminator{1..7,10..12} (10 alike) : Terminator
  Select(1b) : Outport
  wires:
  Logical Operator13/1 -> Select(1b)/1
  1/1 -> Logical Operator13/16, Logical Operator13/15, Logical Operator13/14, Logical Operator13/13, Logical Operator13/12, Logical Operator13/11, Logical Operator13/10, Logical Operator13/8, Logical Operator13/7, Logical Operator13/9
  Bus Selector1/4 -> Logical Operator12/1  "<b12>"
  Bus Selector1/16 -> Terminator12/1  "<b00>"
  Bus Selector1/14 -> Terminator10/1  "<b02>"
  Bus Selector1/12 -> Terminator1/1  "<b04>"
  Bus Selector1/11 -> Terminator3/1  "<b05>"
  Bus Selector1/1 -> Logical Operator10/1  "<b15>"
  Bus Selector1/2 -> Logical Operator11/1  "<b14>"
  Bus Selector1/10 -> Terminator4/1  "<b06>"
  Addr(2B)/1 -> Bus Selector1/1
  Logical Operator12/1 -> Logical Operator13/4
  Bus Selector1/9 -> Terminator5/1  "<b07>"
  Bus Selector1/15 -> Terminator11/1  "<b01>"
  Bus Selector1/13 -> Terminator2/1  "<b03>"
  Bus Selector1/3 -> Logical Operator13/3  "<b13>"
  Logical Operator1/1 -> Logical Operator13/5
  Bus Selector1/5 -> Logical Operator1/1  "<b11>"
  Logical Operator2/1 -> Logical Operator13/6
  Bus Selector1/6 -> Logical Operator2/1  "<b10>"
  Bus Selector1/8 -> Terminator6/1  "<b08>"
  Bus Selector1/7 -> Terminator7/1  "<b09>"
  Logical Operator10/1 -> Logical Operator13/1
  Logical Operator11/1 -> Logical Operator13/2

== system Garbage/<0xFFF6..0xFFF6> ==
  note: Address_Select
  Addr(2B) : Inport
  Bus Selector1 : BusSelector  OutputSignals=b15,b14,b13,b12,b11,b10,b09,b08,b07,b06,b05,b04,b03,b02,b01,b00
  Logical Operator1 : Logic  Operator=NOT  Inputs=1  IconShape=distinctive  AllPortsSameDT=off  OutDataTypeStr=boolean
  Logical Operator12 : Logic  Operator=NOT  Inputs=1  IconShape=distinctive  AllPortsSameDT=off  OutDataTypeStr=boolean
  Logical Operator13 : Logic  Inputs=16  IconShape=distinctive  AllPortsSameDT=off  OutDataTypeStr=boolean
  Select(1b) : Outport
  wires:
  Logical Operator13/1 -> Select(1b)/1
  Addr(2B)/1 -> Bus Selector1/1
  Bus Selector1/15 -> Logical Operator13/15  "<b01>"
  Bus Selector1/7 -> Logical Operator13/7  "<b09>"
  Bus Selector1/13 -> Logical Operator12/1  "<b03>"
  Bus Selector1/11 -> Logical Operator13/11  "<b05>"
  Bus Selector1/9 -> Logical Operator13/9  "<b07>"
  Bus Selector1/6 -> Logical Operator13/6  "<b10>"
  Bus Selector1/8 -> Logical Operator13/8  "<b08>"
  Bus Selector1/3 -> Logical Operator13/3  "<b13>"
  Bus Selector1/10 -> Logical Operator13/10  "<b06>"
  Bus Selector1/4 -> Logical Operator13/4  "<b12>"
  Bus Selector1/14 -> Logical Operator13/14  "<b02>"
  Bus Selector1/12 -> Logical Operator13/12  "<b04>"
  Bus Selector1/16 -> Logical Operator1/1  "<b00>"
  Bus Selector1/5 -> Logical Operator13/5  "<b11>"
  Bus Selector1/1 -> Logical Operator13/1  "<b15>"
  Bus Selector1/2 -> Logical Operator13/2  "<b14>"
  Logical Operator12/1 -> Logical Operator13/13
  Logical Operator1/1 -> Logical Operator13/16

== system Garbage/<0xFFFE..0xFFFE> ==
  note: Address_Select
  Addr(2B) : Inport
  Bus Selector1 : BusSelector  OutputSignals=b15,b14,b13,b12,b11,b10,b09,b08,b07,b06,b05,b04,b03,b02,b01,b00
  Logical Operator12 : Logic  Operator=NOT  Inputs=1  IconShape=distinctive  AllPortsSameDT=off  OutDataTypeStr=boolean
  Logical Operator13 : Logic  Inputs=16  IconShape=distinctive  AllPortsSameDT=off  OutDataTypeStr=boolean
  Select(1b) : Outport
  wires:
  Logical Operator13/1 -> Select(1b)/1
  Addr(2B)/1 -> Bus Selector1/1
  Logical Operator12/1 -> Logical Operator13/16
  Bus Selector1/4 -> Logical Operator13/4  "<b12>"
  Bus Selector1/3 -> Logical Operator13/3  "<b13>"
  Bus Selector1/16 -> Logical Operator12/1  "<b00>"
  Bus Selector1/1 -> Logical Operator13/1  "<b15>"
  Bus Selector1/2 -> Logical Operator13/2  "<b14>"
  Bus Selector1/15 -> Logical Operator13/15  "<b01>"
  Bus Selector1/5 -> Logical Operator13/5  "<b11>"
  Bus Selector1/6 -> Logical Operator13/6  "<b10>"
  Bus Selector1/7 -> Logical Operator13/7  "<b09>"
  Bus Selector1/8 -> Logical Operator13/8  "<b08>"
  Bus Selector1/9 -> Logical Operator13/9  "<b07>"
  Bus Selector1/10 -> Logical Operator13/10  "<b06>"
  Bus Selector1/11 -> Logical Operator13/11  "<b05>"
  Bus Selector1/12 -> Logical Operator13/12  "<b04>"
  Bus Selector1/13 -> Logical Operator13/13  "<b03>"
  Bus Selector1/14 -> Logical Operator13/14  "<b02>"

== system Garbage/Bit_Demultiplexer_1_to_256 ==
  Addr(1B) : Inport
  Data_In(1b) : Inport  Port=2
  1_to_16_Bit_Demultiplexer{,1..16} (17 alike) : Bit_Demultiplexers_Lib/Bit_Demultiplexers_Lib/Bit_Demultiplexer_1_to_16
  Demux1 : Demux  Outputs=16
  Demux17 : Demux  Outputs=8
  Mux : Mux  Inputs=16
  Data_Out(256b) : Outport  OutDataTypeStr=boolean
  wires:
  Mux/1 -> Data_Out(256b)/1
  Demux17/4 -> 1_to_16_Bit_Demultiplexer16/4
  Demux17/3 -> 1_to_16_Bit_Demultiplexer16/3
  Demux17/2 -> 1_to_16_Bit_Demultiplexer16/2
  Demux17/1 -> 1_to_16_Bit_Demultiplexer16/1
  Demux1/16 -> 1_to_16_Bit_Demultiplexer15/5
  Demux1/15 -> 1_to_16_Bit_Demultiplexer14/5
  Demux1/14 -> 1_to_16_Bit_Demultiplexer13/5
  Demux1/13 -> 1_to_16_Bit_Demultiplexer12/5
  Demux1/12 -> 1_to_16_Bit_Demultiplexer11/5
  Demux1/11 -> 1_to_16_Bit_Demultiplexer10/5
  Demux1/10 -> 1_to_16_Bit_Demultiplexer9/5
  Demux1/9 -> 1_to_16_Bit_Demultiplexer8/5
  Demux1/8 -> 1_to_16_Bit_Demultiplexer7/5
  Demux1/7 -> 1_to_16_Bit_Demultiplexer6/5
  Demux1/6 -> 1_to_16_Bit_Demultiplexer5/5
  Demux1/5 -> 1_to_16_Bit_Demultiplexer4/5
  Demux1/4 -> 1_to_16_Bit_Demultiplexer3/5
  Demux1/3 -> 1_to_16_Bit_Demultiplexer2/5
  Demux1/2 -> 1_to_16_Bit_Demultiplexer1/5
  Demux1/1 -> 1_to_16_Bit_Demultiplexer/5
  1_to_16_Bit_Demultiplexer16/1 -> Demux1/1
  Data_In(1b)/1 -> 1_to_16_Bit_Demultiplexer16/5
  1_to_16_Bit_Demultiplexer/1 -> Mux/1
  1_to_16_Bit_Demultiplexer1/1 -> Mux/2
  1_to_16_Bit_Demultiplexer2/1 -> Mux/3
  1_to_16_Bit_Demultiplexer3/1 -> Mux/4
  1_to_16_Bit_Demultiplexer15/1 -> Mux/16
  1_to_16_Bit_Demultiplexer14/1 -> Mux/15
  1_to_16_Bit_Demultiplexer13/1 -> Mux/14
  1_to_16_Bit_Demultiplexer12/1 -> Mux/13
  1_to_16_Bit_Demultiplexer11/1 -> Mux/12
  1_to_16_Bit_Demultiplexer10/1 -> Mux/11
  1_to_16_Bit_Demultiplexer9/1 -> Mux/10
  1_to_16_Bit_Demultiplexer8/1 -> Mux/9
  1_to_16_Bit_Demultiplexer7/1 -> Mux/8
  1_to_16_Bit_Demultiplexer6/1 -> Mux/7
  1_to_16_Bit_Demultiplexer5/1 -> Mux/6
  1_to_16_Bit_Demultiplexer4/1 -> Mux/5
  Demux17/5 -> 1_to_16_Bit_Demultiplexer/1, 1_to_16_Bit_Demultiplexer1/1, 1_to_16_Bit_Demultiplexer2/1, 1_to_16_Bit_Demultiplexer3/1, 1_to_16_Bit_Demultiplexer4/1, 1_to_16_Bit_Demultiplexer5/1, 1_to_16_Bit_Demultiplexer6/1, 1_to_16_Bit_Demultiplexer7/1, 1_to_16_Bit_Demultiplexer8/1, 1_to_16_Bit_Demultiplexer9/1, 1_to_16_Bit_Demultiplexer10/1, 1_to_16_Bit_Demultiplexer11/1, 1_to_16_Bit_Demultiplexer12/1, 1_to_16_Bit_Demultiplexer13/1, 1_to_16_Bit_Demultiplexer14/1, 1_to_16_Bit_Demultiplexer15/1
  Addr(1B)/1 -> Demux17/1
  Demux17/7 -> 1_to_16_Bit_Demultiplexer/3, 1_to_16_Bit_Demultiplexer1/3, 1_to_16_Bit_Demultiplexer2/3, 1_to_16_Bit_Demultiplexer3/3, 1_to_16_Bit_Demultiplexer4/3, 1_to_16_Bit_Demultiplexer5/3, 1_to_16_Bit_Demultiplexer6/3, 1_to_16_Bit_Demultiplexer7/3, 1_to_16_Bit_Demultiplexer8/3, 1_to_16_Bit_Demultiplexer9/3, 1_to_16_Bit_Demultiplexer10/3, 1_to_16_Bit_Demultiplexer11/3, 1_to_16_Bit_Demultiplexer12/3, 1_to_16_Bit_Demultiplexer13/3, 1_to_16_Bit_Demultiplexer14/3, 1_to_16_Bit_Demultiplexer15/3
  Demux17/6 -> 1_to_16_Bit_Demultiplexer/2, 1_to_16_Bit_Demultiplexer1/2, 1_to_16_Bit_Demultiplexer2/2, 1_to_16_Bit_Demultiplexer3/2, 1_to_16_Bit_Demultiplexer4/2, 1_to_16_Bit_Demultiplexer5/2, 1_to_16_Bit_Demultiplexer6/2, 1_to_16_Bit_Demultiplexer7/2, 1_to_16_Bit_Demultiplexer8/2, 1_to_16_Bit_Demultiplexer9/2, 1_to_16_Bit_Demultiplexer10/2, 1_to_16_Bit_Demultiplexer11/2, 1_to_16_Bit_Demultiplexer12/2, 1_to_16_Bit_Demultiplexer13/2, 1_to_16_Bit_Demultiplexer14/2, 1_to_16_Bit_Demultiplexer15/2
  Demux17/8 -> 1_to_16_Bit_Demultiplexer/4, 1_to_16_Bit_Demultiplexer1/4, 1_to_16_Bit_Demultiplexer2/4, 1_to_16_Bit_Demultiplexer3/4, 1_to_16_Bit_Demultiplexer4/4, 1_to_16_Bit_Demultiplexer5/4, 1_to_16_Bit_Demultiplexer6/4, 1_to_16_Bit_Demultiplexer7/4, 1_to_16_Bit_Demultiplexer8/4, 1_to_16_Bit_Demultiplexer9/4, 1_to_16_Bit_Demultiplexer10/4, 1_to_16_Bit_Demultiplexer11/4, 1_to_16_Bit_Demultiplexer12/4, 1_to_16_Bit_Demultiplexer13/4, 1_to_16_Bit_Demultiplexer14/4, 1_to_16_Bit_Demultiplexer15/4
